simulink model "AcasXuClosedLoop_all"  (Simulink 10.0)
settings: Solver=VariableStepAuto  StartTime=0.0  StopTime=20  RelTol=1e-3
libraries: neural x14040, simulink x2
(part 19 of 534)

== system AcasXuClosedLoop_all/networks/Networks_m_4/AcasXu_3_4/Layer 5 ==
  "a{4} " : Inport  PortDimensions=50
      mask Display: e = 0.06;c1 = [120 230 180]/255;c2 = ([120 230 180]+0)/(256+128);c3 = ([120 230 180]+128)/(256+128);patch([0 1 1 0],[0 0 1 1],c1);patch([0 1 1 1-e 1-e 0+e],[0 0 1 1-e 0+e 0+e],c2);patch([0 0 1 1-e 0+e 0+e],[0 1 1 1-e 1-e 0+e],c3);plot([0 1 1 0 0],[0 0 1 1 0]);
  Delays 1 : Subsystem
      mask Display: e = 0.06;c1 = [120 150 230]/255;c2 = ([120 150 230]+0)/383;c3 = ([120 150 230]+128)/383;patch([0 1 1 0],[0 0 1 1],c1);patch([0 1 1 1-e 1-e 0+e],[0 0 1 1-e 0+e 0+e],c2);patch([0 0 1 1-e 0+e 0+e],[0 1 1 1-e 1-e 0+e],c3);plot([0 1 1 0 0],[0 0 1 1 0]);disp('0');
  "LW{5,4}" : Subsystem
      mask Display: e = 0.06;c1 = [120 150 230]/255;c2 = ([120 150 230]+0)/383;c3 = ([120 150 230]+128)/383;patch([0 1 1 0],[0 0 1 1],c1);patch([0 1 1 1-e 1-e 0+e],[0 0 1 1-e 0+e 0+e],c2);patch([0 0 1 1-e 0+e 0+e],[0 1 1 1-e 1-e 0+e],c3);plot([0 1 1 0 0],[0 0 1 1 0]);disp('W');
  "b{5}" : Constant  Value=[1.4490899999999999891997504164464771747589111328125;-1.4936000000000000387245790989254601299762725830078125;-1.49167000000000005144329406903125345706939697265625;-3.37314999999999987068122209166176617145538330078125;-0.400770000000000015116796703296131454408168792724609375;0.275085999999999997189803480068803764879703521728515625;0.66838900000000001089262013920233584940433502197265625;-2.935529999999999972715158946812152862548828125;-1.9079800000000000093081098384573124349117279052734375;-2.69432999999999989171328707016073167324066162109375;2.202030000000000153903556565637700259685516357421875;-1.7417499999999999094058011905872263014316558837890625;1.3679699999999999082689328133710660040378570556640625;-3.99077999999999999403144101961515843868255615234375;0.65461100000000005394440449890680611133575439453125;1.1150700000000000056132876125047914683818817138671875;-1.0728899999999998993160943427938036620616912841796875;0.19173899999999999277378037731978110969066619873046875;-0.7763839999999999630375668857595883309841156005859375;-1.9096900000000001096367441277834586799144744873046875;0.414737999999999995548449760462972335517406463623046875;1.8134300000000000974154090727097354829311370849609375;0.446747999999999978459896965432562865316867828369140625;-0.4394970000000000265316657532821409404277801513671875;-0.0279925000000000000210942374678779742680490016937255859375;-0.98748899999999995014121623171376995742321014404296875;-1.372100000000000097344354799133725464344024658203125;1.0688400000000000122923893286497332155704498291015625;-1.62661999999999995480948200565762817859649658203125;0.75358599999999997809396745651611126959323883056640625;0.19033400000000000318323145620524883270263671875;2.329590000000000049595882956054992973804473876953125;2.042229999999999989768184605054557323455810546875;-0.681599999999999983657517077517695724964141845703125;0.21860299999999999176480969254043884575366973876953125;-0.6634949999999999459276978086563758552074432373046875;0.078978300000000001279687467103940434753894805908203125;1.218599999999999905497816143906675279140472412109375;1.7972200000000000397193389289896003901958465576171875;0.037543100000000002858246972436973010189831256866455078125;-3.1881099999999999994315658113919198513031005859375;0.379817000000000015713652601334615610539913177490234375;0.76774799999999998600941353288362734019756317138671875;-0.11585399999999999864908062363610952161252498626708984375;-1.58790999999999993264054864994250237941741943359375;-0.33601300000000000611777295489446260035037994384765625;-0.91279399999999999426592012241599150002002716064453125;1.48285000000000000142108547152020037174224853515625;0.253029000000000003911537760359351523220539093017578125;-1.375389999999999890434310145792551338672637939453125]
      mask Display: e = 0.06;c1 = [120 150 230]/255;c2 = ([120 150 230]+0)/383;c3 = ([120 150 230]+128)/383;patch([0 1 1 0],[0 0 1 1],c1);patch([0 1 1 1-e 1-e 0+e],[0 0 1 1-e 0+e 0+e],c2);patch([0 0 1 1-e 0+e 0+e],[0 1 1 1-e 1-e 0+e],c3);plot([0 1 1 0 0],[0 0 1 1 0]);disp('b');
  netsum : Sum  Inputs=2  SaturateOnIntegerOverflow=on  InputSameDT=on  OutDataTypeStr=Inherit: Same as first input
      mask Display: e = 0.06;c1 = [120 150 230]/255;c2 = ([120 150 230]+0)/383;c3 = ([120 150 230]+128)/383;patch([0 1 1 0],[0 0 1 1],c1);patch([0 1 1 1-e 1-e 0+e],[0 0 1 1-e 0+e 0+e],c2);patch([0 0 1 1-e 0+e 0+e],[0 1 1 1-e 1-e 0+e],c3);plot([0 1 1 0 0],[0 0 1 1 0]);disp('+');
  poslin : neural/Transfer Functions/poslin
      settings: SourceProductName=Deep Learning Toolbox  SourceProductBaseCode=NN
  "a{5}" : Outport  InitialOutput=[0;0;0;0;0;0;0;0;0;0;0;0;0;0;0;0;0;0;0;0;0;0;0;0;0;0;0;0;0;0;0;0;0;0;0;0;0;0;0;0;0;0;0;0;0;0;0;0;0;0]
      mask Display: e = 0.06;c1 = [120 230 180]/255;c2 = ([120 230 180]+0)/(256+128);c3 = ([120 230 180]+128)/(256+128);patch([0 1 1 0],[0 0 1 1],c1);patch([0 1 1 1-e 1-e 0+e],[0 0 1 1-e 0+e 0+e],c2);patch([0 0 1 1-e 0+e 0+e],[0 1 1 1-e 1-e 0+e],c3);plot([0 1 1 0 0],[0 0 1 1 0]);
  wires:
  poslin/1 -> "a{5}"/1
  netsum/1 -> poslin/1
  "b{5}"/1 -> netsum/2
  "LW{5,4}"/1("lz{5,4}") -> netsum/1
  Delays 1/1("pd{5,4}") -> "LW{5,4}"/1("ad{5,4}")
  "a{4} "/1 -> Delays 1/1("p{5}")

== system AcasXuClosedLoop_all/networks/Networks_m_4/AcasXu_3_4/Layer 6 ==
  "a{5} " : Inport  PortDimensions=50
      mask Display: e = 0.06;c1 = [120 230 180]/255;c2 = ([120 230 180]+0)/(256+128);c3 = ([120 230 180]+128)/(256+128);patch([0 1 1 0],[0 0 1 1],c1);patch([0 1 1 1-e 1-e 0+e],[0 0 1 1-e 0+e 0+e],c2);patch([0 0 1 1-e 0+e 0+e],[0 1 1 1-e 1-e 0+e],c3);plot([0 1 1 0 0],[0 0 1 1 0]);
  Delays 1 : Subsystem
      mask Display: e = 0.06;c1 = [120 150 230]/255;c2 = ([120 150 230]+0)/383;c3 = ([120 150 230]+128)/383;patch([0 1 1 0],[0 0 1 1],c1);patch([0 1 1 1-e 1-e 0+e],[0 0 1 1-e 0+e 0+e],c2);patch([0 0 1 1-e 0+e 0+e],[0 1 1 1-e 1-e 0+e],c3);plot([0 1 1 0 0],[0 0 1 1 0]);disp('0');
  "LW{6,5}" : Subsystem
      mask Display: e = 0.06;c1 = [120 150 230]/255;c2 = ([120 150 230]+0)/383;c3 = ([120 150 230]+128)/383;patch([0 1 1 0],[0 0 1 1],c1);patch([0 1 1 1-e 1-e 0+e],[0 0 1 1-e 0+e 0+e],c2);patch([0 0 1 1-e 0+e 0+e],[0 1 1 1-e 1-e 0+e],c3);plot([0 1 1 0 0],[0 0 1 1 0]);disp('W');
  "b{6}" : Constant  Value=[-0.54986100000000004417444188220542855560779571533203125;0.1197529999999999983373299983213655650615692138671875;-1.7450900000000000300559577226522378623485565185546875;-0.051296500000000001817657135916306287981569766998291015625;0.2438349999999999961897145794864627532660961151123046875;-1.0193700000000001093525270334794186055660247802734375;0.78492499999999998383515276145772077143192291259765625;-1.331350000000000033395508580724708735942840576171875;1.029549999999999965183405947755090892314910888671875;-0.27535100000000001241318159372895024716854095458984375;-0.209496999999999988784082916026818566024303436279296875;0.0196731999999999983275156267836791812442243099212646484375;0.15212700000000001221422962771612219512462615966796875;-0.59908200000000000340349970429087989032268524169921875;0.310972000000000026176394385402090847492218017578125;-0.5571040000000000436131131209549494087696075439453125;-0.354453999999999991299404200617573224008083343505859375;-0.3631349999999999855759824640699662268161773681640625;0.05090340000000000142410527814718079753220081329345703125;0.5594959999999999933351091385702602565288543701171875;-0.032265700000000001324007570246976683847606182098388671875;-0.0270350000000000000033306690738754696212708950042724609375;0.300810999999999995058175272788503207266330718994140625;0.3671459999999999723740984336473047733306884765625;0.45297500000000001652011860642232932150363922119140625;0.289443999999999979078069145543850027024745941162109375;-2.9526599999999998402699930011294782161712646484375;0.28581000000000000849098569233319722115993499755859375;0.134151999999999993473664972043479792773723602294921875;-0.09278889999999999360635882794667850248515605926513671875;0.155073999999999989629628771581337787210941314697265625;0.0155467999999999995086596982218907214701175689697265625;-0.286609999999999975894837689338601194322109222412109375;-0.0001768259999999999905646863584962602544692344963550567626953125;-0.64645099999999999784705551064689643681049346923828125;1.444080000000000030269120543380267918109893798828125;0.35234799999999999453592636200482957065105438232421875;0.60375400000000001288213979933061636984348297119140625;0.42682100000000000594724269831203855574131011962890625;0.86949799999999999311484089048462919890880584716796875;0.478937000000000001609379296496626920998096466064453125;0.281440999999999996727950701824738644063472747802734375;-0.43791400000000002545874622228438965976238250732421875;0.00919550000000000054833915186236481531523168087005615234375;-0.327890000000000014779288903810083866119384765625;0.164125999999999994116706147906370460987091064453125;-0.2313219999999999998419042412933777086436748504638671875;0.53194900000000000517275111633352935314178466796875;0.35422799999999998732391759403981268405914306640625;-2.163670000000000204209982257452793419361114501953125]
      mask Display: e = 0.06;c1 = [120 150 230]/255;c2 = ([120 150 230]+0)/383;c3 = ([120 150 230]+128)/383;patch([0 1 1 0],[0 0 1 1],c1);patch([0 1 1 1-e 1-e 0+e],[0 0 1 1-e 0+e 0+e],c2);patch([0 0 1 1-e 0+e 0+e],[0 1 1 1-e 1-e 0+e],c3);plot([0 1 1 0 0],[0 0 1 1 0]);disp('b');
  netsum : Sum  Inputs=2  SaturateOnIntegerOverflow=on  InputSameDT=on  OutDataTypeStr=Inherit: Same as first input
      mask Display: e = 0.06;c1 = [120 150 230]/255;c2 = ([120 150 230]+0)/383;c3 = ([120 150 230]+128)/383;patch([0 1 1 0],[0 0 1 1],c1);patch([0 1 1 1-e 1-e 0+e],[0 0 1 1-e 0+e 0+e],c2);patch([0 0 1 1-e 0+e 0+e],[0 1 1 1-e 1-e 0+e],c3);plot([0 1 1 0 0],[0 0 1 1 0]);disp('+');
  poslin : neural/Transfer Functions/poslin
      settings: SourceProductName=Deep Learning Toolbox  SourceProductBaseCode=NN
  "a{6}" : Outport  InitialOutput=[0;0;0;0;0;0;0;0;0;0;0;0;0;0;0;0;0;0;0;0;0;0;0;0;0;0;0;0;0;0;0;0;0;0;0;0;0;0;0;0;0;0;0;0;0;0;0;0;0;0]
      mask Display: e = 0.06;c1 = [120 230 180]/255;c2 = ([120 230 180]+0)/(256+128);c3 = ([120 230 180]+128)/(256+128);patch([0 1 1 0],[0 0 1 1],c1);patch([0 1 1 1-e 1-e 0+e],[0 0 1 1-e 0+e 0+e],c2);patch([0 0 1 1-e 0+e 0+e],[0 1 1 1-e 1-e 0+e],c3);plot([0 1 1 0 0],[0 0 1 1 0]);
  wires:
  poslin/1 -> "a{6}"/1
  netsum/1 -> poslin/1
  "b{6}"/1 -> netsum/2
  "LW{6,5}"/1("lz{6,5}") -> netsum/1
  Delays 1/1("pd{6,5}") -> "LW{6,5}"/1("ad{6,5}")
  "a{5} "/1 -> Delays 1/1("p{6}")

== system AcasXuClosedLoop_all/networks/Networks_m_4/AcasXu_3_4/Layer 7 ==
  "a{6} " : Inport  PortDimensions=50
      mask Display: e = 0.06;c1 = [120 230 180]/255;c2 = ([120 230 180]+0)/(256+128);c3 = ([120 230 180]+128)/(256+128);patch([0 1 1 0],[0 0 1 1],c1);patch([0 1 1 1-e 1-e 0+e],[0 0 1 1-e 0+e 0+e],c2);patch([0 0 1 1-e 0+e 0+e],[0 1 1 1-e 1-e 0+e],c3);plot([0 1 1 0 0],[0 0 1 1 0]);
  Delays 1 : Subsystem
      mask Display: e = 0.06;c1 = [120 150 230]/255;c2 = ([120 150 230]+0)/383;c3 = ([120 150 230]+128)/383;patch([0 1 1 0],[0 0 1 1],c1);patch([0 1 1 1-e 1-e 0+e],[0 0 1 1-e 0+e 0+e],c2);patch([0 0 1 1-e 0+e 0+e],[0 1 1 1-e 1-e 0+e],c3);plot([0 1 1 0 0],[0 0 1 1 0]);disp('0');
  "LW{7,6}" : Subsystem
      mask Display: e = 0.06;c1 = [120 150 230]/255;c2 = ([120 150 230]+0)/383;c3 = ([120 150 230]+128)/383;patch([0 1 1 0],[0 0 1 1],c1);patch([0 1 1 1-e 1-e 0+e],[0 0 1 1-e 0+e 0+e],c2);patch([0 0 1 1-e 0+e 0+e],[0 1 1 1-e 1-e 0+e],c3);plot([0 1 1 0 0],[0 0 1 1 0]);disp('W');
  "b{7}" : Constant  Value=[-0.0207768000000000015170531497687989030964672565460205078125;0.018318199999999999760813551574756274931132793426513671875;-0.01982810000000000127950983142000040970742702484130859375;0.018992900000000000060307314697638503275811672210693359375;-0.0180815999999999997560617970293606049381196498870849609375]
      mask Display: e = 0.06;c1 = [120 150 230]/255;c2 = ([120 150 230]+0)/383;c3 = ([120 150 230]+128)/383;patch([0 1 1 0],[0 0 1 1],c1);patch([0 1 1 1-e 1-e 0+e],[0 0 1 1-e 0+e 0+e],c2);patch([0 0 1 1-e 0+e 0+e],[0 1 1 1-e 1-e 0+e],c3);plot([0 1 1 0 0],[0 0 1 1 0]);disp('b');
  netsum : Sum  Inputs=2  SaturateOnIntegerOverflow=on  InputSameDT=on  OutDataTypeStr=Inherit: Same as first input
      mask Display: e = 0.06;c1 = [120 150 230]/255;c2 = ([120 150 230]+0)/383;c3 = ([120 150 230]+128)/383;patch([0 1 1 0],[0 0 1 1],c1);patch([0 1 1 1-e 1-e 0+e],[0 0 1 1-e 0+e 0+e],c2);patch([0 0 1 1-e 0+e 0+e],[0 1 1 1-e 1-e 0+e],c3);plot([0 1 1 0 0],[0 0 1 1 0]);disp('+');
  purelin : neural/Transfer Functions/purelin
      settings: SourceProductName=Deep Learning Toolbox
  "a{7}" : Outport  InitialOutput=[0;0;0;0;0]
      mask Display: e = 0.06;c1 = [120 230 180]/255;c2 = ([120 230 180]+0)/(256+128);c3 = ([120 230 180]+128)/(256+128);patch([0 1 1 0],[0 0 1 1],c1);patch([0 1 1 1-e 1-e 0+e],[0 0 1 1-e 0+e 0+e],c2);patch([0 0 1 1-e 0+e 0+e],[0 1 1 1-e 1-e 0+e],c3);plot([0 1 1 0 0],[0 0 1 1 0]);
  wires:
  purelin/1 -> "a{7}"/1
  netsum/1 -> purelin/1
  "b{7}"/1 -> netsum/2
  "LW{7,6}"/1("lz{7,6}") -> netsum/1
  Delays 1/1("pd{7,6}") -> "LW{7,6}"/1("ad{7,6}")
  "a{6} "/1 -> Delays 1/1("p{7}")

== system AcasXuClosedLoop_all/networks/Networks_m_4/AcasXu_3_4/Process Input 1 ==
  x : Inport  PortDimensions=5
      mask Display: e = 0.06;c1 = [120 230 180]/255;c2 = ([120 230 180]+0)/(256+128);c3 = ([120 230 180]+128)/(256+128);patch([0 1 1 0],[0 0 1 1],c1);patch([0 1 1 1-e 1-e 0+e],[0 0 1 1-e 0+e 0+e],c2);patch([0 0 1 1-e 0+e 0+e],[0 1 1 1-e 1-e 0+e],c3);plot([0 1 1 0 0],[0 0 1 1 0]);
  p : Outport  PortDimensions=5
      mask Display: e = 0.06;c1 = [120 230 180]/255;c2 = ([120 230 180]+0)/(256+128);c3 = ([120 230 180]+128)/(256+128);patch([0 1 1 0],[0 0 1 1],c1);patch([0 1 1 1-e 1-e 0+e],[0 0 1 1-e 0+e 0+e],c2);patch([0 0 1 1-e 0+e 0+e],[0 1 1 1-e 1-e 0+e],c3);plot([0 1 1 0 0],[0 0 1 1 0]);
  wires:
  x/1 -> p/1

== system AcasXuClosedLoop_all/networks/Networks_m_4/AcasXu_3_4/Process Output 1 ==
  a : Inport  PortDimensions=5
      mask Display: e = 0.06;c1 = [120 230 180]/255;c2 = ([120 230 180]+0)/(256+128);c3 = ([120 230 180]+128)/(256+128);patch([0 1 1 0],[0 0 1 1],c1);patch([0 1 1 1-e 1-e 0+e],[0 0 1 1-e 0+e 0+e],c2);patch([0 0 1 1-e 0+e 0+e],[0 1 1 1-e 1-e 0+e],c3);plot([0 1 1 0 0],[0 0 1 1 0]);
  y : Outport  PortDimensions=5
      mask Display: e = 0.06;c1 = [120 230 180]/255;c2 = ([120 230 180]+0)/(256+128);c3 = ([120 230 180]+128)/(256+128);patch([0 1 1 0],[0 0 1 1],c1);patch([0 1 1 1-e 1-e 0+e],[0 0 1 1-e 0+e 0+e],c2);patch([0 0 1 1-e 0+e 0+e],[0 1 1 1-e 1-e 0+e],c3);plot([0 1 1 0 0],[0 0 1 1 0]);
  wires:
  a/1 -> y/1

== system AcasXuClosedLoop_all/networks/Networks_m_4/AcasXu_4_4/Layer 1 ==
  "p{1}" : Inport  PortDimensions=5
      mask Display: e = 0.06;c1 = [120 230 180]/255;c2 = ([120 230 180]+0)/(256+128);c3 = ([120 230 180]+128)/(256+128);patch([0 1 1 0],[0 0 1 1],c1);patch([0 1 1 1-e 1-e 0+e],[0 0 1 1-e 0+e 0+e],c2);patch([0 0 1 1-e 0+e 0+e],[0 1 1 1-e 1-e 0+e],c3);plot([0 1 1 0 0],[0 0 1 1 0]);
  Delays 1 : Subsystem
      mask Display: e = 0.06;c1 = [120 150 230]/255;c2 = ([120 150 230]+0)/383;c3 = ([120 150 230]+128)/383;patch([0 1 1 0],[0 0 1 1],c1);patch([0 1 1 1-e 1-e 0+e],[0 0 1 1-e 0+e 0+e],c2);patch([0 0 1 1-e 0+e 0+e],[0 1 1 1-e 1-e 0+e],c3);plot([0 1 1 0 0],[0 0 1 1 0]);disp('0');
  "IW{1,1}" : Subsystem
      mask Display: e = 0.06;c1 = [120 150 230]/255;c2 = ([120 150 230]+0)/383;c3 = ([120 150 230]+128)/383;patch([0 1 1 0],[0 0 1 1],c1);patch([0 1 1 1-e 1-e 0+e],[0 0 1 1-e 0+e 0+e],c2);patch([0 0 1 1-e 0+e 0+e],[0 1 1 1-e 1-e 0+e],c3);plot([0 1 1 0 0],[0 0 1 1 0]);disp('W');
  "b{1}" : Constant  Value=[0.1853569999999999939888084554695524275302886962890625;0.161592000000000013404388710114290006458759307861328125;0.09378789999999999349444834706446272321045398712158203125;-0.0209424999999999993105515017077777883969247341156005859375;-0.04625089999999999756941093664863728918135166168212890625;0.5106699999999999572963815808179788291454315185546875;-0.34228599999999997915978156015626154839992523193359375;-0.0075299700000000004129763198079672292806208133697509765625;0.53827199999999997270805351945455186069011688232421875;0.1806230000000000057713833712114137597382068634033203125;0.28324300000000002253131015095277689397335052490234375;-0.157652999999999987590371119949850253760814666748046875;0.15598400000000001153210860138642601668834686279296875;0.07062970000000000359019480811184621416032314300537109375;0.413837999999999983646858936481294222176074981689453125;0.02629449999999999842970055397017858922481536865234375;0.0677284999999999970388131487197824753820896148681640625;0.27142699999999997384492189667071215808391571044921875;0.426536999999999999477751089216326363384723663330078125;-0.1210050000000000014477308241112041287124156951904296875;0.321653000000000022229329488254734314978122711181640625;-0.13089899999999998758681840627104975283145904541015625;-0.1516349999999999920152760068958741612732410430908203125;-0.093359899999999995667820940070669166743755340576171875;-0.43019499999999999406696815640316344797611236572265625;-0.05967419999999999669082484388127340935170650482177734375;-0.10303700000000000358557628032940556295216083526611328125;-0.281403000000000014235723710953607223927974700927734375;0.156569000000000013717027513848734088242053985595703125;0.28647699999999998166089199003181420266628265380859375;-0.14882200000000000983391146291978657245635986328125;-0.324587000000000014399148540178430266678333282470703125;-0.67928399999999999891997504164464771747589111328125;-0.10523000000000000409006162271907669492065906524658203125;-0.25771399999999999863575794734060764312744140625;-0.2361540000000000028013147357341949827969074249267578125;-0.0652363999999999999435118525070720352232456207275390625;-0.611563999999999996504129740060307085514068603515625;-0.047255199999999997206856505727046169340610504150390625;-0.445643999999999984584775347684626467525959014892578125;0.10648100000000000620037354792657424695789813995361328125;0.1885360000000000091358032250354881398379802703857421875;-0.30424499999999998767208353456226177513599395751953125;-0.299263000000000001232791646543773822486400604248046875;0.0041381100000000003991118546764482744038105010986328125;0.39901500000000000856203996590920723974704742431640625;-0.289302000000000003598898956624907441437244415283203125;0.1397500000000000131006316905768471769988536834716796875;0.1421820000000000028261837314857984893023967742919921875;0.6036040000000000294022584057529456913471221923828125]
      mask Display: e = 0.06;c1 = [120 150 230]/255;c2 = ([120 150 230]+0)/383;c3 = ([120 150 230]+128)/383;patch([0 1 1 0],[0 0 1 1],c1);patch([0 1 1 1-e 1-e 0+e],[0 0 1 1-e 0+e 0+e],c2);patch([0 0 1 1-e 0+e 0+e],[0 1 1 1-e 1-e 0+e],c3);plot([0 1 1 0 0],[0 0 1 1 0]);disp('b');
  netsum : Sum  Inputs=2  SaturateOnIntegerOverflow=on  InputSameDT=on  OutDataTypeStr=Inherit: Same as first input
      mask Display: e = 0.06;c1 = [120 150 230]/255;c2 = ([120 150 230]+0)/383;c3 = ([120 150 230]+128)/383;patch([0 1 1 0],[0 0 1 1],c1);patch([0 1 1 1-e 1-e 0+e],[0 0 1 1-e 0+e 0+e],c2);patch([0 0 1 1-e 0+e 0+e],[0 1 1 1-e 1-e 0+e],c3);plot([0 1 1 0 0],[0 0 1 1 0]);disp('+');
  poslin : neural/Transfer Functions/poslin
      settings: SourceProductName=Deep Learning Toolbox  SourceProductBaseCode=NN
  "a{1}" : Outport  InitialOutput=[0;0;0;0;0;0;0;0;0;0;0;0;0;0;0;0;0;0;0;0;0;0;0;0;0;0;0;0;0;0;0;0;0;0;0;0;0;0;0;0;0;0;0;0;0;0;0;0;0;0]
      mask Display: e = 0.06;c1 = [120 230 180]/255;c2 = ([120 230 180]+0)/(256+128);c3 = ([120 230 180]+128)/(256+128);patch([0 1 1 0],[0 0 1 1],c1);patch([0 1 1 1-e 1-e 0+e],[0 0 1 1-e 0+e 0+e],c2);patch([0 0 1 1-e 0+e 0+e],[0 1 1 1-e 1-e 0+e],c3);plot([0 1 1 0 0],[0 0 1 1 0]);
  wires:
  poslin/1 -> "a{1}"/1
  netsum/1 -> poslin/1
  "b{1}"/1 -> netsum/2
  "IW{1,1}"/1("iz{1,1}") -> netsum/1
  Delays 1/1("pd{1,1}") -> "IW{1,1}"/1("pd{1,1}")
  "p{1}"/1 -> Delays 1/1("p{1}")

== system AcasXuClosedLoop_all/networks/Networks_m_4/AcasXu_4_4/Layer 2 ==
  "a{1} " : Inport  PortDimensions=50
      mask Display: e = 0.06;c1 = [120 230 180]/255;c2 = ([120 230 180]+0)/(256+128);c3 = ([120 230 180]+128)/(256+128);patch([0 1 1 0],[0 0 1 1],c1);patch([0 1 1 1-e 1-e 0+e],[0 0 1 1-e 0+e 0+e],c2);patch([0 0 1 1-e 0+e 0+e],[0 1 1 1-e 1-e 0+e],c3);plot([0 1 1 0 0],[0 0 1 1 0]);
  Delays 1 : Subsystem
      mask Display: e = 0.06;c1 = [120 150 230]/255;c2 = ([120 150 230]+0)/383;c3 = ([120 150 230]+128)/383;patch([0 1 1 0],[0 0 1 1],c1);patch([0 1 1 1-e 1-e 0+e],[0 0 1 1-e 0+e 0+e],c2);patch([0 0 1 1-e 0+e 0+e],[0 1 1 1-e 1-e 0+e],c3);plot([0 1 1 0 0],[0 0 1 1 0]);disp('0');
  "LW{2,1}" : Subsystem
      mask Display: e = 0.06;c1 = [120 150 230]/255;c2 = ([120 150 230]+0)/383;c3 = ([120 150 230]+128)/383;patch([0 1 1 0],[0 0 1 1],c1);patch([0 1 1 1-e 1-e 0+e],[0 0 1 1-e 0+e 0+e],c2);patch([0 0 1 1-e 0+e 0+e],[0 1 1 1-e 1-e 0+e],c3);plot([0 1 1 0 0],[0 0 1 1 0]);disp('W');
  "b{2}" : Constant  Value=[-0.7588369999999999837569930605241097509860992431640625;0.452266000000000001346478484265389852225780487060546875;0.630314999999999958646412778762169182300567626953125;-0.01103580000000000012339018695683989790268242359161376953125;-0.485578999999999982861709213466383516788482666015625;-0.87777000000000005019984428145107813179492950439453125;-0.01450919999999999987494447850622236728668212890625;0.27304600000000001092104184863273985683917999267578125;-0.01156800000000000015754064719430971308611333370208740234375;-0.11960300000000000097966079692923813126981258392333984375;-0.01800090000000000023394619574901298619806766510009765625;-0.75817800000000001858779796748422086238861083984375;-0.00832216000000000032110758496628477587364614009857177734375;-0.09419180000000000607673911190431681461632251739501953125;-0.01060400000000000057809312892231901059858500957489013671875;-0.082037799999999994060573271781322546303272247314453125;0.7729639999999999844249032321386039257049560546875;-0.3003270000000000106155084722558967769145965576171875;-0.760116000000000013869794202037155628204345703125;-0.10269799999999999762234637046276475302875041961669921875;-1.172239999999999948698814478120766580104827880859375;-0.414665000000000005808686864838819019496440887451171875;0.77132500000000003836930773104541003704071044921875;-0.6109269999999999978257392285740934312343597412109375;-0.026329499999999998738342554815972107462584972381591796875;-0.3368430000000000035242919693700969219207763671875;0.90736899999999998112087951085413806140422821044921875;-1.12708999999999992525090419803746044635772705078125;-0.454239000000000003876010623571346513926982879638671875;-0.01181800000000000037958525211934102117083966732025146484375;-0.00632191999999999997006394636400727904401719570159912109375;-0.0151576999999999996460164908285150886513292789459228515625;-0.0183930999999999990335286526033087284304201602935791015625;-0.0127746999999999999442223952428321354091167449951171875;0.090778999999999998582467242158600129187107086181640625;-0.48900799999999999823074858795735053718090057373046875;-0.0210333000000000013007817045718184090219438076019287109375;-0.23611199999999998855315652690478600561618804931640625;0.0179617000000000005821565451924470835365355014801025390625;1.0619799999999999240429815472452901303768157958984375;1.074079999999999923687710179365240037441253662109375;-0.1818140000000000033875124927362776361405849456787109375;0.316321000000000018825829783963854424655437469482421875;-0.241773999999999988919086035821237601339817047119140625;0.3829759999999999831032937436248175799846649169921875;-0.1402819999999999900364144878039951436221599578857421875;-1.2630600000000000715516534910420887172222137451171875;0.55671999999999999264588268488296307623386383056640625;0.98400399999999998978950088712736032903194427490234375;-0.00774478999999999966064034850887765060178935527801513671875]
      mask Display: e = 0.06;c1 = [120 150 230]/255;c2 = ([120 150 230]+0)/383;c3 = ([120 150 230]+128)/383;patch([0 1 1 0],[0 0 1 1],c1);patch([0 1 1 1-e 1-e 0+e],[0 0 1 1-e 0+e 0+e],c2);patch([0 0 1 1-e 0+e 0+e],[0 1 1 1-e 1-e 0+e],c3);plot([0 1 1 0 0],[0 0 1 1 0]);disp('b');
  netsum : Sum  Inputs=2  SaturateOnIntegerOverflow=on  InputSameDT=on  OutDataTypeStr=Inherit: Same as first input
      mask Display: e = 0.06;c1 = [120 150 230]/255;c2 = ([120 150 230]+0)/383;c3 = ([120 150 230]+128)/383;patch([0 1 1 0],[0 0 1 1],c1);patch([0 1 1 1-e 1-e 0+e],[0 0 1 1-e 0+e 0+e],c2);patch([0 0 1 1-e 0+e 0+e],[0 1 1 1-e 1-e 0+e],c3);plot([0 1 1 0 0],[0 0 1 1 0]);disp('+');
  poslin : neural/Transfer Functions/poslin
      settings: SourceProductName=Deep Learning Toolbox  SourceProductBaseCode=NN
  "a{2}" : Outport  InitialOutput=[0;0;0;0;0;0;0;0;0;0;0;0;0;0;0;0;0;0;0;0;0;0;0;0;0;0;0;0;0;0;0;0;0;0;0;0;0;0;0;0;0;0;0;0;0;0;0;0;0;0]
      mask Display: e = 0.06;c1 = [120 230 180]/255;c2 = ([120 230 180]+0)/(256+128);c3 = ([120 230 180]+128)/(256+128);patch([0 1 1 0],[0 0 1 1],c1);patch([0 1 1 1-e 1-e 0+e],[0 0 1 1-e 0+e 0+e],c2);patch([0 0 1 1-e 0+e 0+e],[0 1 1 1-e 1-e 0+e],c3);plot([0 1 1 0 0],[0 0 1 1 0]);
  wires:
  poslin/1 -> "a{2}"/1
  netsum/1 -> poslin/1
  "b{2}"/1 -> netsum/2
  "LW{2,1}"/1("lz{2,1}") -> netsum/1
  Delays 1/1("pd{2,1}") -> "LW{2,1}"/1("ad{2,1}")
  "a{1} "/1 -> Delays 1/1("p{2}")

== system AcasXuClosedLoop_all/networks/Networks_m_4/AcasXu_4_4/Layer 3 ==
  "a{2} " : Inport  PortDimensions=50
      mask Display: e = 0.06;c1 = [120 230 180]/255;c2 = ([120 230 180]+0)/(256+128);c3 = ([120 230 180]+128)/(256+128);patch([0 1 1 0],[0 0 1 1],c1);patch([0 1 1 1-e 1-e 0+e],[0 0 1 1-e 0+e 0+e],c2);patch([0 0 1 1-e 0+e 0+e],[0 1 1 1-e 1-e 0+e],c3);plot([0 1 1 0 0],[0 0 1 1 0]);
  Delays 1 : Subsystem
      mask Display: e = 0.06;c1 = [120 150 230]/255;c2 = ([120 150 230]+0)/383;c3 = ([120 150 230]+128)/383;patch([0 1 1 0],[0 0 1 1],c1);patch([0 1 1 1-e 1-e 0+e],[0 0 1 1-e 0+e 0+e],c2);patch([0 0 1 1-e 0+e 0+e],[0 1 1 1-e 1-e 0+e],c3);plot([0 1 1 0 0],[0 0 1 1 0]);disp('0');
  "LW{3,2}" : Subsystem
      mask Display: e = 0.06;c1 = [120 150 230]/255;c2 = ([120 150 230]+0)/383;c3 = ([120 150 230]+128)/383;patch([0 1 1 0],[0 0 1 1],c1);patch([0 1 1 1-e 1-e 0+e],[0 0 1 1-e 0+e 0+e],c2);patch([0 0 1 1-e 0+e 0+e],[0 1 1 1-e 1-e 0+e],c3);plot([0 1 1 0 0],[0 0 1 1 0]);disp('W');
  "b{3}" : Constant  Value=[-0.0258787000000000010080380974386571324430406093597412109375;-0.12835900000000000087396756498492322862148284912109375;-0.30833100000000002172129143218626268208026885986328125;-2.05928999999999984282794684986583888530731201171875;1.533910000000000106723518911167047917842864990234375;0.075847300000000006381384309861459769308567047119140625;-1.618279999999999940740735837607644498348236083984375;0.51613900000000001444533381800283677875995635986328125;-3.817480000000000206483719011885114014148712158203125;-1.54183000000000003382183422218076884746551513671875;-1.429580000000000072901684688986279070377349853515625;-0.56528900000000004144595777688664384186267852783203125;-0.1893219999999999902939862295170314610004425048828125;-1.1526300000000000434141611549421213567256927490234375;-0.0080841000000000003133937553911891882307827472686767578125;-0.062033999999999998864463890413389890454709529876708984375;-1.1482200000000000184030568561865948140621185302734375;0.241794000000000008920864047468057833611965179443359375;-0.7712440000000000406288336307625286281108856201171875;-2.08729999999999993320898283855058252811431884765625;-1.612109999999999931930005914182402193546295166015625;-1.3550299999999999567279473922098986804485321044921875;0.453259000000000022989610215518041513860225677490234375;-1.1341200000000000169819713846663944423198699951171875;-0.031291300000000001058086951388759189285337924957275390625;-0.54478499999999996372679333944688551127910614013671875;0.678779000000000021231016944511793553829193115234375;0.35429699999999997306332488733460195362567901611328125;-1.1835199999999999054267618703306652605533599853515625;0.08215350000000000429967172976830624975264072418212890625;-0.046713999999999998580246796109349816106259822845458984375;-0.71354600000000001358557710773311555385589599609375;0.9661750000000000060396132539608515799045562744140625;-1.2595300000000000384403620046214200556278228759765625;0.10561800000000000354649642986260005272924900054931640625;-0.2317740000000000077928774544488987885415554046630859375;0.1743449999999999999733546474089962430298328399658203125;0.0466482000000000007755573960821493528783321380615234375;0.64660200000000001008260142043582163751125335693359375;-0.343278000000000027558400006455485709011554718017578125;-0.0534567999999999987625898256737855263054370880126953125;-0.362449999999999994404475955889211036264896392822265625;1.4021999999999998909316900608246214687824249267578125;-0.0152318000000000002003286425633632461540400981903076171875;1.4956300000000000149924517245381139218807220458984375;1.266129999999999977688958097132854163646697998046875;-0.0319035000000000013020695632803835906088352203369140625;-0.3644330000000000069348971010185778141021728515625;-1.701189999999999979962694851565174758434295654296875;0.2642189999999999816537865626742132008075714111328125]
      mask Display: e = 0.06;c1 = [120 150 230]/255;c2 = ([120 150 230]+0)/383;c3 = ([120 150 230]+128)/383;patch([0 1 1 0],[0 0 1 1],c1);patch([0 1 1 1-e 1-e 0+e],[0 0 1 1-e 0+e 0+e],c2);patch([0 0 1 1-e 0+e 0+e],[0 1 1 1-e 1-e 0+e],c3);plot([0 1 1 0 0],[0 0 1 1 0]);disp('b');
  netsum : Sum  Inputs=2  SaturateOnIntegerOverflow=on  InputSameDT=on  OutDataTypeStr=Inherit: Same as first input
      mask Display: e = 0.06;c1 = [120 150 230]/255;c2 = ([120 150 230]+0)/383;c3 = ([120 150 230]+128)/383;patch([0 1 1 0],[0 0 1 1],c1);patch([0 1 1 1-e 1-e 0+e],[0 0 1 1-e 0+e 0+e],c2);patch([0 0 1 1-e 0+e 0+e],[0 1 1 1-e 1-e 0+e],c3);plot([0 1 1 0 0],[0 0 1 1 0]);disp('+');
  poslin : neural/Transfer Functions/poslin
      settings: SourceProductName=Deep Learning Toolbox  SourceProductBaseCode=NN
  "a{3}" : Outport  InitialOutput=[0;0;0;0;0;0;0;0;0;0;0;0;0;0;0;0;0;0;0;0;0;0;0;0;0;0;0;0;0;0;0;0;0;0;0;0;0;0;0;0;0;0;0;0;0;0;0;0;0;0]
      mask Display: e = 0.06;c1 = [120 230 180]/255;c2 = ([120 230 180]+0)/(256+128);c3 = ([120 230 180]+128)/(256+128);patch([0 1 1 0],[0 0 1 1],c1);patch([0 1 1 1-e 1-e 0+e],[0 0 1 1-e 0+e 0+e],c2);patch([0 0 1 1-e 0+e 0+e],[0 1 1 1-e 1-e 0+e],c3);plot([0 1 1 0 0],[0 0 1 1 0]);
  wires:
  poslin/1 -> "a{3}"/1
  netsum/1 -> poslin/1
  "b{3}"/1 -> netsum/2
  "LW{3,2}"/1("lz{3,2}") -> netsum/1
  Delays 1/1("pd{3,2}") -> "LW{3,2}"/1("ad{3,2}")
  "a{2} "/1 -> Delays 1/1("p{3}")

== system AcasXuClosedLoop_all/networks/Networks_m_4/AcasXu_4_4/Layer 4 ==
  "a{3} " : Inport  PortDimensions=50
      mask Display: e = 0.06;c1 = [120 230 180]/255;c2 = ([120 230 180]+0)/(256+128);c3 = ([120 230 180]+128)/(256+128);patch([0 1 1 0],[0 0 1 1],c1);patch([0 1 1 1-e 1-e 0+e],[0 0 1 1-e 0+e 0+e],c2);patch([0 0 1 1-e 0+e 0+e],[0 1 1 1-e 1-e 0+e],c3);plot([0 1 1 0 0],[0 0 1 1 0]);
  Delays 1 : Subsystem
      mask Display: e = 0.06;c1 = [120 150 230]/255;c2 = ([120 150 230]+0)/383;c3 = ([120 150 230]+128)/383;patch([0 1 1 0],[0 0 1 1],c1);patch([0 1 1 1-e 1-e 0+e],[0 0 1 1-e 0+e 0+e],c2);patch([0 0 1 1-e 0+e 0+e],[0 1 1 1-e 1-e 0+e],c3);plot([0 1 1 0 0],[0 0 1 1 0]);disp('0');
  "LW{4,3}" : Subsystem
      mask Display: e = 0.06;c1 = [120 150 230]/255;c2 = ([120 150 230]+0)/383;c3 = ([120 150 230]+128)/383;patch([0 1 1 0],[0 0 1 1],c1);patch([0 1 1 1-e 1-e 0+e],[0 0 1 1-e 0+e 0+e],c2);patch([0 0 1 1-e 0+e 0+e],[0 1 1 1-e 1-e 0+e],c3);plot([0 1 1 0 0],[0 0 1 1 0]);disp('W');
  "b{4}" : Constant  Value=[-0.439242999999999994553689930398832075297832489013671875;-1.3779999999999998916422327965847216546535491943359375;-0.21750700000000000589039927945123054087162017822265625;-0.656552000000000024471091819577850401401519775390625;0.038018099999999999116795379450195468962192535400390625;0.14212700000000000333244543071486987173557281494140625;-1.9346699999999998897948216836084611713886260986328125;-0.29171300000000000007815970093361102044582366943359375;-0.013350199999999999567190656080128974281251430511474609375;-1.720909999999999939745976007543504238128662109375;-0.32441799999999998416200241990736685693264007568359375;-1.63182999999999989171328707016073167324066162109375;-1.2422299999999999453592636200482957065105438232421875;0.32354300000000002501820972611312754452228546142578125;1.03024000000000004462208380573429167270660400390625;0.677202999999999999403144101961515843868255615234375;1.050450000000000105870867628254927694797515869140625;-0.69108899999999995333865854263422079384326934814453125;-2.3966300000000000380850906367413699626922607421875;-1.6163000000000000699884594723698683083057403564453125;-0.48753999999999997339017454578424803912639617919921875;-4.14848000000000016740386854507960379123687744140625;-2.752829999999999888160573391360230743885040283203125;1.2115199999999999302957576219341717660427093505859375;-0.62237500000000001154631945610162802040576934814453125;0.08680209999999999315178911274415440857410430908203125;-0.5518229999999999524362692682188935577869415283203125;-0.32209399999999999142374917937559075653553009033203125;-2.40796000000000010032863428932614624500274658203125;0.057577200000000001989253206602370482869446277618408203125;-2.298620000000000107576170194079168140888214111328125;-1.4327099999999999280220208675018511712551116943359375;-0.60511099999999995446842149249278008937835693359375;0.27355099999999998860999994576559402048587799072265625;-0.1508649999999999991029397961028735153377056121826171875;1.0237700000000000688515910951537080109119415283203125;-0.93717600000000000903810359886847436428070068359375;-0.0155834000000000007235545496087070205248892307281494140625;-0.470009999999999983355536414819653145968914031982421875;-0.042988800000000000622879525735697825439274311065673828125;1.582689999999999930224703348358161747455596923828125;2.0980199999999999960209606797434389591217041015625;-0.2209309999999999885034185354015789926052093505859375;0.337417999999999995708321876008994877338409423828125;0.1598380000000000078497208733097068034112453460693359375;0.490310999999999996834532112188753671944141387939453125;-0.11610099999999999587085852681411779485642910003662109375;-0.51208299999999995488764170659123919904232025146484375;-1.2019100000000000338928884957567788660526275634765625;-0.62458100000000005280753612169064581394195556640625]
      mask Display: e = 0.06;c1 = [120 150 230]/255;c2 = ([120 150 230]+0)/383;c3 = ([120 150 230]+128)/383;patch([0 1 1 0],[0 0 1 1],c1);patch([0 1 1 1-e 1-e 0+e],[0 0 1 1-e 0+e 0+e],c2);patch([0 0 1 1-e 0+e 0+e],[0 1 1 1-e 1-e 0+e],c3);plot([0 1 1 0 0],[0 0 1 1 0]);disp('b');
  netsum : Sum  Inputs=2  SaturateOnIntegerOverflow=on  InputSameDT=on  OutDataTypeStr=Inherit: Same as first input
      mask Display: e = 0.06;c1 = [120 150 230]/255;c2 = ([120 150 230]+0)/383;c3 = ([120 150 230]+128)/383;patch([0 1 1 0],[0 0 1 1],c1);patch([0 1 1 1-e 1-e 0+e],[0 0 1 1-e 0+e 0+e],c2);patch([0 0 1 1-e 0+e 0+e],[0 1 1 1-e 1-e 0+e],c3);plot([0 1 1 0 0],[0 0 1 1 0]);disp('+');
  poslin : neural/Transfer Functions/poslin
      settings: SourceProductName=Deep Learning Toolbox  SourceProductBaseCode=NN
  "a{4}" : Outport  InitialOutput=[0;0;0;0;0;0;0;0;0;0;0;0;0;0;0;0;0;0;0;0;0;0;0;0;0;0;0;0;0;0;0;0;0;0;0;0;0;0;0;0;0;0;0;0;0;0;0;0;0;0]
      mask Display: e = 0.06;c1 = [120 230 180]/255;c2 = ([120 230 180]+0)/(256+128);c3 = ([120 230 180]+128)/(256+128);patch([0 1 1 0],[0 0 1 1],c1);patch([0 1 1 1-e 1-e 0+e],[0 0 1 1-e 0+e 0+e],c2);patch([0 0 1 1-e 0+e 0+e],[0 1 1 1-e 1-e 0+e],c3);plot([0 1 1 0 0],[0 0 1 1 0]);
  wires:
  poslin/1 -> "a{4}"/1
  netsum/1 -> poslin/1
  "b{4}"/1 -> netsum/2
  "LW{4,3}"/1("lz{4,3}") -> netsum/1
  Delays 1/1("pd{4,3}") -> "LW{4,3}"/1("ad{4,3}")
  "a{3} "/1 -> Delays 1/1("p{4}")

== system AcasXuClosedLoop_all/networks/Networks_m_4/AcasXu_4_4/Layer 5 ==
  "a{4} " : Inport  PortDimensions=50
      mask Display: e = 0.06;c1 = [120 230 180]/255;c2 = ([120 230 180]+0)/(256+128);c3 = ([120 230 180]+128)/(256+128);patch([0 1 1 0],[0 0 1 1],c1);patch([0 1 1 1-e 1-e 0+e],[0 0 1 1-e 0+e 0+e],c2);patch([0 0 1 1-e 0+e 0+e],[0 1 1 1-e 1-e 0+e],c3);plot([0 1 1 0 0],[0 0 1 1 0]);
  Delays 1 : Subsystem
      mask Display: e = 0.06;c1 = [120 150 230]/255;c2 = ([120 150 230]+0)/383;c3 = ([120 150 230]+128)/383;patch([0 1 1 0],[0 0 1 1],c1);patch([0 1 1 1-e 1-e 0+e],[0 0 1 1-e 0+e 0+e],c2);patch([0 0 1 1-e 0+e 0+e],[0 1 1 1-e 1-e 0+e],c3);plot([0 1 1 0 0],[0 0 1 1 0]);disp('0');
  "LW{5,4}" : Subsystem
      mask Display: e = 0.06;c1 = [120 150 230]/255;c2 = ([120 150 230]+0)/383;c3 = ([120 150 230]+128)/383;patch([0 1 1 0],[0 0 1 1],c1);patch([0 1 1 1-e 1-e 0+e],[0 0 1 1-e 0+e 0+e],c2);patch([0 0 1 1-e 0+e 0+e],[0 1 1 1-e 1-e 0+e],c3);plot([0 1 1 0 0],[0 0 1 1 0]);disp('W');
  "b{5}" : Constant  Value=[-1.109690000000000065227823142777197062969207763671875;-0.6209189999999999987068122209166176617145538330078125;0.391311000000000019927171024392009712755680084228515625;-0.383645999999999987029042358699371106922626495361328125;-0.274716000000000015734968883407418616116046905517578125;0.634387999999999951938889353186823427677154541015625;0.74609899999999995667820940070669166743755340576171875;1.203770000000000006679101716144941747188568115234375;0.11113699999999999967759123364885454066097736358642578125;-2.291819999999999968309793985099531710147857666015625;-1.8992599999999999482724888366647064685821533203125;-0.896093000000000028393287720973603427410125732421875;0.5493710000000000537312416781787760555744171142578125;-0.72295600000000004303757350498926825821399688720703125;-2.246519999999999850359699848922900855541229248046875;-2.322719999999999895834434937569312751293182373046875;3.10897999999999985476506481063552200794219970703125;0.775317999999999951654672258882783353328704833984375;0.5036140000000000060964566728216595947742462158203125;0.95876799999999995360866478222305886447429656982421875;0.01237839999999999947288831236846817773766815662384033203125;0.89011300000000004306599521441967226564884185791015625;0.377107000000000025519142354823998175561428070068359375;1.943750000000000088817841970012523233890533447265625;-1.2657199999999999562305674771778285503387451171875;1.105259999999999909192638369859196245670318603515625;1.200159999999999893560698183136992156505584716796875;0.1936859999999999970565767171137849800288677215576171875;-0.378800999999999998824051772317034192383289337158203125;0.6764559999999999462261257576756179332733154296875;1.1008400000000000407140987590537406504154205322265625;0.1314470000000000082795992284445674158632755279541015625;0.4360749999999999904076730672386474907398223876953125;-1.2892699999999999160849029067321680486202239990234375;-0.93927700000000002855671255019842647016048431396484375;-4.1347100000000001074340616469271481037139892578125;0.840716000000000018843593352357856929302215576171875;1.2266300000000001091393642127513885498046875;1.5043200000000001015365569401183165609836578369140625;0.1709730000000000138538069904825533740222454071044921875;-0.55356700000000003125677494608680717647075653076171875;1.1156800000000000050448534238967113196849822998046875;-0.03244149999999999811617357181603438220918178558349609375;0.1625669999999999892903446152558899484574794769287109375;-1.009560000000000012931877790833823382854461669921875;-4.78953999999999968650854498264379799365997314453125;0.37843999999999999861444166526780463755130767822265625;-1.743700000000000027711166694643907248973846435546875;-0.0173015000000000009061640326990527682937681674957275390625;-0.326043000000000027238655775363440625369548797607421875]
      mask Display: e = 0.06;c1 = [120 150 230]/255;c2 = ([120 150 230]+0)/383;c3 = ([120 150 230]+128)/383;patch([0 1 1 0],[0 0 1 1],c1);patch([0 1 1 1-e 1-e 0+e],[0 0 1 1-e 0+e 0+e],c2);patch([0 0 1 1-e 0+e 0+e],[0 1 1 1-e 1-e 0+e],c3);plot([0 1 1 0 0],[0 0 1 1 0]);disp('b');
  netsum : Sum  Inputs=2  SaturateOnIntegerOverflow=on  InputSameDT=on  OutDataTypeStr=Inherit: Same as first input
      mask Display: e = 0.06;c1 = [120 150 230]/255;c2 = ([120 150 230]+0)/383;c3 = ([120 150 230]+128)/383;patch([0 1 1 0],[0 0 1 1],c1);patch([0 1 1 1-e 1-e 0+e],[0 0 1 1-e 0+e 0+e],c2);patch([0 0 1 1-e 0+e 0+e],[0 1 1 1-e 1-e 0+e],c3);plot([0 1 1 0 0],[0 0 1 1 0]);disp('+');
  poslin : neural/Transfer Functions/poslin
      settings: SourceProductName=Deep Learning Toolbox  SourceProductBaseCode=NN
  "a{5}" : Outport  InitialOutput=[0;0;0;0;0;0;0;0;0;0;0;0;0;0;0;0;0;0;0;0;0;0;0;0;0;0;0;0;0;0;0;0;0;0;0;0;0;0;0;0;0;0;0;0;0;0;0;0;0;0]
      mask Display: e = 0.06;c1 = [120 230 180]/255;c2 = ([120 230 180]+0)/(256+128);c3 = ([120 230 180]+128)/(256+128);patch([0 1 1 0],[0 0 1 1],c1);patch([0 1 1 1-e 1-e 0+e],[0 0 1 1-e 0+e 0+e],c2);patch([0 0 1 1-e 0+e 0+e],[0 1 1 1-e 1-e 0+e],c3);plot([0 1 1 0 0],[0 0 1 1 0]);
  wires:
  poslin/1 -> "a{5}"/1
  netsum/1 -> poslin/1
  "b{5}"/1 -> netsum/2
  "LW{5,4}"/1("lz{5,4}") -> netsum/1
  Delays 1/1("pd{5,4}") -> "LW{5,4}"/1("ad{5,4}")
  "a{4} "/1 -> Delays 1/1("p{5}")

== system AcasXuClosedLoop_all/networks/Networks_m_4/AcasXu_4_4/Layer 6 ==
  "a{5} " : Inport  PortDimensions=50
      mask Display: e = 0.06;c1 = [120 230 180]/255;c2 = ([120 230 180]+0)/(256+128);c3 = ([120 230 180]+128)/(256+128);patch([0 1 1 0],[0 0 1 1],c1);patch([0 1 1 1-e 1-e 0+e],[0 0 1 1-e 0+e 0+e],c2);patch([0 0 1 1-e 0+e 0+e],[0 1 1 1-e 1-e 0+e],c3);plot([0 1 1 0 0],[0 0 1 1 0]);
  Delays 1 : Subsystem
      mask Display: e = 0.06;c1 = [120 150 230]/255;c2 = ([120 150 230]+0)/383;c3 = ([120 150 230]+128)/383;patch([0 1 1 0],[0 0 1 1],c1);patch([0 1 1 1-e 1-e 0+e],[0 0 1 1-e 0+e 0+e],c2);patch([0 0 1 1-e 0+e 0+e],[0 1 1 1-e 1-e 0+e],c3);plot([0 1 1 0 0],[0 0 1 1 0]);disp('0');
  "LW{6,5}" : Subsystem
      mask Display: e = 0.06;c1 = [120 150 230]/255;c2 = ([120 150 230]+0)/383;c3 = ([120 150 230]+128)/383;patch([0 1 1 0],[0 0 1 1],c1);patch([0 1 1 1-e 1-e 0+e],[0 0 1 1-e 0+e 0+e],c2);patch([0 0 1 1-e 0+e 0+e],[0 1 1 1-e 1-e 0+e],c3);plot([0 1 1 0 0],[0 0 1 1 0]);disp('W');
  "b{6}" : Constant  Value=[0.3377689999999999859170429772348143160343170166015625;0.155476000000000003087308186877635307610034942626953125;0.359283000000000019014123608940280973911285400390625;-1.0577799999999999425170926770078949630260467529296875;-0.133438000000000001055155962603748776018619537353515625;1.137259999999999937614347800263203680515289306640625;0.59142700000000003601741127567947842180728912353515625;-0.0236826000000000015666135055880658910609781742095947265625;-0.1254939999999999944435558063560165464878082275390625;0.418642000000000014114931445874390192329883575439453125;0.63417599999999996196464735476183705031871795654296875;0.238373000000000001552535877635818906128406524658203125;0.306059000000000025476509790678392164409160614013671875;0.901297000000000014807710613240487873554229736328125;0.196566999999999991732835269431234337389469146728515625;0.52427900000000005054090479461592622101306915283203125;-0.322589999999999987867482786896289326250553131103515625;-0.1438669999999999948858686593666789121925830841064453125;-0.2831099999999999727862132203881628811359405517578125;0.424420000000000019468870959826745092868804931640625;1.253649999999999931077354631270281970500946044921875;0.6945259999999999767084091217839159071445465087890625;0.4006009999999999848796505830250680446624755859375;-0.00898357999999999969065189731054488220252096652984619140625;-1.2850200000000000510880227011512033641338348388671875;0.4171679999999999832738240002072416245937347412109375;0.4020449999999999857180910112219862639904022216796875;0.70626500000000003165467887811246328055858612060546875;0.202139000000000013113066188452648930251598358154296875;0.6499829999999999774473735669744201004505157470703125;0.47118599999999999372590764323831535875797271728515625;0.3007239999999999913171677690115757286548614501953125;1.4790200000000000013500311979441903531551361083984375;1.8509100000000000552091705685597844421863555908203125;0.7147769999999999956941110212937928736209869384765625;0.79708400000000001472955091230687685310840606689453125;-2.3291100000000000136424205265939235687255859375;0.6032159999999999744346723673515953123569488525390625;0.2049809999999999965414332336877123452723026275634765625;0.25337399999999998811972545809112489223480224609375;-0.544178000000000050562221076688729226589202880859375;-0.549509000000000025210056264768354594707489013671875;0.452091999999999993864463476711534895002841949462890625;0.322465999999999974878761577201657928526401519775390625;0.9052540000000000031121771826292388141155242919921875;-0.031071700000000000707611746975089772604405879974365234375;-1.4779500000000000969890834312536753714084625244140625;0.49440899999999998737365558554301969707012176513671875;0.46014100000000002221867134721833281219005584716796875;-0.33063500000000001222133505507372319698333740234375]
      mask Display: e = 0.06;c1 = [120 150 230]/255;c2 = ([120 150 230]+0)/383;c3 = ([120 150 230]+128)/383;patch([0 1 1 0],[0 0 1 1],c1);patch([0 1 1 1-e 1-e 0+e],[0 0 1 1-e 0+e 0+e],c2);patch([0 0 1 1-e 0+e 0+e],[0 1 1 1-e 1-e 0+e],c3);plot([0 1 1 0 0],[0 0 1 1 0]);disp('b');
  netsum : Sum  Inputs=2  SaturateOnIntegerOverflow=on  InputSameDT=on  OutDataTypeStr=Inherit: Same as first input
      mask Display: e = 0.06;c1 = [120 150 230]/255;c2 = ([120 150 230]+0)/383;c3 = ([120 150 230]+128)/383;patch([0 1 1 0],[0 0 1 1],c1);patch([0 1 1 1-e 1-e 0+e],[0 0 1 1-e 0+e 0+e],c2);patch([0 0 1 1-e 0+e 0+e],[0 1 1 1-e 1-e 0+e],c3);plot([0 1 1 0 0],[0 0 1 1 0]);disp('+');
  poslin : neural/Transfer Functions/poslin
      settings: SourceProductName=Deep Learning Toolbox  SourceProductBaseCode=NN
  "a{6}" : Outport  InitialOutput=[0;0;0;0;0;0;0;0;0;0;0;0;0;0;0;0;0;0;0;0;0;0;0;0;0;0;0;0;0;0;0;0;0;0;0;0;0;0;0;0;0;0;0;0;0;0;0;0;0;0]
      mask Display: e = 0.06;c1 = [120 230 180]/255;c2 = ([120 230 180]+0)/(256+128);c3 = ([120 230 180]+128)/(256+128);patch([0 1 1 0],[0 0 1 1],c1);patch([0 1 1 1-e 1-e 0+e],[0 0 1 1-e 0+e 0+e],c2);patch([0 0 1 1-e 0+e 0+e],[0 1 1 1-e 1-e 0+e],c3);plot([0 1 1 0 0],[0 0 1 1 0]);
  wires:
  poslin/1 -> "a{6}"/1
  netsum/1 -> poslin/1
  "b{6}"/1 -> netsum/2
  "LW{6,5}"/1("lz{6,5}") -> netsum/1
  Delays 1/1("pd{6,5}") -> "LW{6,5}"/1("ad{6,5}")
  "a{5} "/1 -> Delays 1/1("p{6}")

== system AcasXuClosedLoop_all/networks/Networks_m_4/AcasXu_4_4/Layer 7 ==
  "a{6} " : Inport  PortDimensions=50
      mask Display: e = 0.06;c1 = [120 230 180]/255;c2 = ([120 230 180]+0)/(256+128);c3 = ([120 230 180]+128)/(256+128);patch([0 1 1 0],[0 0 1 1],c1);patch([0 1 1 1-e 1-e 0+e],[0 0 1 1-e 0+e 0+e],c2);patch([0 0 1 1-e 0+e 0+e],[0 1 1 1-e 1-e 0+e],c3);plot([0 1 1 0 0],[0 0 1 1 0]);
  Delays 1 : Subsystem
      mask Display: e = 0.06;c1 = [120 150 230]/255;c2 = ([120 150 230]+0)/383;c3 = ([120 150 230]+128)/383;patch([0 1 1 0],[0 0 1 1],c1);patch([0 1 1 1-e 1-e 0+e],[0 0 1 1-e 0+e 0+e],c2);patch([0 0 1 1-e 0+e 0+e],[0 1 1 1-e 1-e 0+e],c3);plot([0 1 1 0 0],[0 0 1 1 0]);disp('0');
  "LW{7,6}" : Subsystem
      mask Display: e = 0.06;c1 = [120 150 230]/255;c2 = ([120 150 230]+0)/383;c3 = ([120 150 230]+128)/383;patch([0 1 1 0],[0 0 1 1],c1);patch([0 1 1 1-e 1-e 0+e],[0 0 1 1-e 0+e 0+e],c2);patch([0 0 1 1-e 0+e 0+e],[0 1 1 1-e 1-e 0+e],c3);plot([0 1 1 0 0],[0 0 1 1 0]);disp('W');
  "b{7}" : Constant  Value=[-0.02058290000000000119495524586454848758876323699951171875;-0.0182620999999999998608668505539753823541104793548583984375;0.0183730999999999998484323526781736291013658046722412109375;-0.0181383999999999989682919476763345301151275634765625;0.018219699999999998396571498915363918058574199676513671875]
      mask Display: e = 0.06;c1 = [120 150 230]/255;c2 = ([120 150 230]+0)/383;c3 = ([120 150 230]+128)/383;patch([0 1 1 0],[0 0 1 1],c1);patch([0 1 1 1-e 1-e 0+e],[0 0 1 1-e 0+e 0+e],c2);patch([0 0 1 1-e 0+e 0+e],[0 1 1 1-e 1-e 0+e],c3);plot([0 1 1 0 0],[0 0 1 1 0]);disp('b');
  netsum : Sum  Inputs=2  SaturateOnIntegerOverflow=on  InputSameDT=on  OutDataTypeStr=Inherit: Same as first input
      mask Display: e = 0.06;c1 = [120 150 230]/255;c2 = ([120 150 230]+0)/383;c3 = ([120 150 230]+128)/383;patch([0 1 1 0],[0 0 1 1],c1);patch([0 1 1 1-e 1-e 0+e],[0 0 1 1-e 0+e 0+e],c2);patch([0 0 1 1-e 0+e 0+e],[0 1 1 1-e 1-e 0+e],c3);plot([0 1 1 0 0],[0 0 1 1 0]);disp('+');
  purelin : neural/Transfer Functions/purelin
      settings: SourceProductName=Deep Learning Toolbox
  "a{7}" : Outport  InitialOutput=[0;0;0;0;0]
      mask Display: e = 0.06;c1 = [120 230 180]/255;c2 = ([120 230 180]+0)/(256+128);c3 = ([120 230 180]+128)/(256+128);patch([0 1 1 0],[0 0 1 1],c1);patch([0 1 1 1-e 1-e 0+e],[0 0 1 1-e 0+e 0+e],c2);patch([0 0 1 1-e 0+e 0+e],[0 1 1 1-e 1-e 0+e],c3);plot([0 1 1 0 0],[0 0 1 1 0]);
  wires:
  purelin/1 -> "a{7}"/1
  netsum/1 -> purelin/1
  "b{7}"/1 -> netsum/2
  "LW{7,6}"/1("lz{7,6}") -> netsum/1
  Delays 1/1("pd{7,6}") -> "LW{7,6}"/1("ad{7,6}")
  "a{6} "/1 -> Delays 1/1("p{7}")

== system AcasXuClosedLoop_all/networks/Networks_m_4/AcasXu_4_4/Process Input 1 ==
  x : Inport  PortDimensions=5
      mask Display: e = 0.06;c1 = [120 230 180]/255;c2 = ([120 230 180]+0)/(256+128);c3 = ([120 230 180]+128)/(256+128);patch([0 1 1 0],[0 0 1 1],c1);patch([0 1 1 1-e 1-e 0+e],[0 0 1 1-e 0+e 0+e],c2);patch([0 0 1 1-e 0+e 0+e],[0 1 1 1-e 1-e 0+e],c3);plot([0 1 1 0 0],[0 0 1 1 0]);
  p : Outport  PortDimensions=5
      mask Display: e = 0.06;c1 = [120 230 180]/255;c2 = ([120 230 180]+0)/(256+128);c3 = ([120 230 180]+128)/(256+128);patch([0 1 1 0],[0 0 1 1],c1);patch([0 1 1 1-e 1-e 0+e],[0 0 1 1-e 0+e 0+e],c2);patch([0 0 1 1-e 0+e 0+e],[0 1 1 1-e 1-e 0+e],c3);plot([0 1 1 0 0],[0 0 1 1 0]);
  wires:
  x/1 -> p/1
